simulink model "ROV_Simulation"  (R2024b) (continued)
== system ROV_Simulation/Recreate 6 DOF ref == (continued)
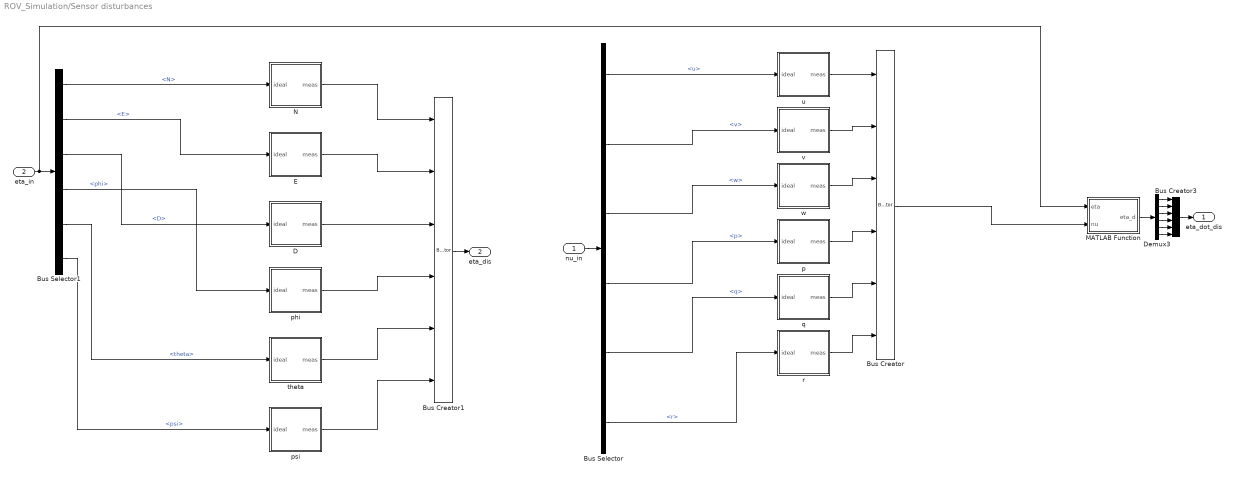
== system ROV_Simulation/Sensor disturbances ==
  nu_in : Inport
  eta_in : Inport  Port=2
  Bus Creator : BusCreator  Inputs=6  OutDataTypeStr=Bus: nu
      settings: InheritFromInputs=off
  Bus Creator1 : BusCreator  Inputs=6  OutDataTypeStr=Bus: eta
      settings: InheritFromInputs=off
  Bus Creator3 : BusCreator  Inputs=6  OutDataTypeStr=Bus: eta_d
      settings: InheritFromInputs=off
  Bus Selector : BusSelector  OutputSignals=u,v,w,p,q,r
  Bus Selector1 : BusSelector  OutputSignals=N,E,D,phi,theta,psi
  D : Subsystem
  Demux3 : Demux  Outputs=6
  E : Subsystem
  MATLAB Function : MATLAB Function  TreatAsAtomicUnit=on
  N : Subsystem
  p : Subsystem
  phi : Subsystem
  psi : Subsystem
  q : Subsystem
  r : Subsystem
  theta : Subsystem
  u : Subsystem
  v : Subsystem
  w : Subsystem
  eta_dot_dis : Outport
  eta_dis : Outport  Port=2
  wires:
  Bus Selector/6 -> r/1(ideal)  "<r>"
  Bus Selector/3 -> w/1(ideal)  "<w>"
  eta_in/1 -> MATLAB Function/1(eta), Bus Selector1/1
  Bus Selector1/2 -> E/1(ideal)  "<E>"
  Bus Selector1/3 -> D/1(ideal)  "<D>"
  Bus Selector1/4 -> phi/1(ideal)  "<phi>"
  Bus Selector1/1 -> N/1(ideal)  "<N>"
  Bus Selector/4 -> p/1(ideal)  "<p>"
  Bus Selector/2 -> v/1(ideal)  "<v>"
  Bus Selector1/6 -> psi/1(ideal)  "<psi>"
  nu_in/1 -> Bus Selector/1
  Bus Selector/5 -> q/1(ideal)  "<q>"
  Bus Selector1/5 -> theta/1(ideal)  "<theta>"
  Bus Selector/1 -> u/1(ideal)  "<u>"
  Bus Creator/1 -> MATLAB Function/2(nu)
  D/1(meas) -> Bus Creator1/3
  phi/1(meas) -> Bus Creator1/4
  theta/1(meas) -> Bus Creator1/5
  psi/1(meas) -> Bus Creator1/6
  N/1(meas) -> Bus Creator1/1
  E/1(meas) -> Bus Creator1/2
  Bus Creator1/1 -> eta_dis/1
  u/1(meas) -> Bus Creator/1
  v/1(meas) -> Bus Creator/2
  w/1(meas) -> Bus Creator/3
  p/1(meas) -> Bus Creator/4
  q/1(meas) -> Bus Creator/5
  r/1(meas) -> Bus Creator/6
  MATLAB Function/1(eta_d) -> Demux3/1
  Demux3/1 -> Bus Creator3/1
  Demux3/5 -> Bus Creator3/5
  Demux3/2 -> Bus Creator3/2
  Bus Creator3/1 -> eta_dot_dis/1
  Demux3/3 -> Bus Creator3/3
  Demux3/4 -> Bus Creator3/4
  Demux3/6 -> Bus Creator3/6

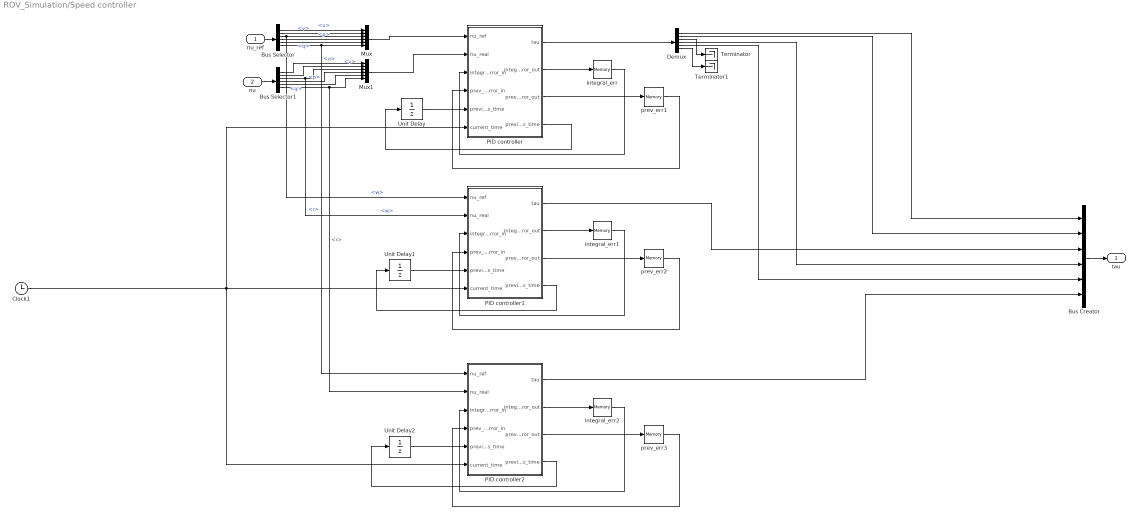
== system ROV_Simulation/Speed controller  ==
  nu_ref : Inport
  nu : Inport  Port=2
  Bus Creator : BusCreator  Inputs=6  OutDataTypeStr=Bus: tau
  Bus Selector : BusSelector  OutputSignals=u,v,w,p,q,r
  Bus Selector1 : BusSelector  OutputSignals=u,v,w,p,q,r
  Clock1 : Clock
  Demux : Demux  Outputs=6
  Mux : Mux  Inputs=6
  Mux1 : Mux  Inputs=6
  PID controller : MATLAB Function  TreatAsAtomicUnit=on
  PID controller1 : MATLAB Function  TreatAsAtomicUnit=on
  PID controller2 : MATLAB Function  TreatAsAtomicUnit=on
  Terminator : Terminator
  Terminator1 : Terminator
  Unit Delay : UnitDelay  SampleTime=-1  HasFrameUpgradeWarning=on
  Unit Delay1 : UnitDelay  SampleTime=-1  HasFrameUpgradeWarning=on
  Unit Delay2 : UnitDelay  SampleTime=-1  HasFrameUpgradeWarning=on
  integral_err : Memory
  integral_err1 : Memory
  integral_err2 : Memory
  prev_err1 : Memory
  prev_err2 : Memory
  prev_err3 : Memory
  tau : Outport
  wires:
  PID controller/1(tau) -> Demux/1
  PID controller/2(integral_error_out) -> integral_err/1
  PID controller/3(prev_error_out) -> prev_err1/1
  prev_err1/1 -> PID controller/4(prev_error_in)
  integral_err/1 -> PID controller/3(integral_error_in)
  Bus Creator/1 -> tau/1
  Demux/1 -> Bus Creator/1
  Demux/2 -> Bus Creator/2
  Demux/4 -> Bus Creator/4
  Demux/5 -> Bus Creator/5
  nu_ref/1 -> Bus Selector/1
  Mux/1 -> PID controller/1(nu_ref)
  nu/1 -> Bus Selector1/1
  Mux1/1 -> PID controller/2(nu_real)
  Unit Delay/1 -> PID controller/5("previous_time ")
  PID controller/4(previous_time) -> Unit Delay/1
  Bus Selector/1 -> Mux/1  "<u>"
  Bus Selector/2 -> Mux/2  "<v>"
  Bus Selector/4 -> Mux/4  "<p>"
  Bus Selector/5 -> Mux/5  "<q>"
  Bus Selector1/1 -> Mux1/1  "<u>"
  Bus Selector1/2 -> Mux1/2  "<v>"
  Bus Selector1/4 -> Mux1/4  "<p>"
  Bus Selector1/5 -> Mux1/5  "<q>"
  Bus Selector1/3 -> Mux1/3, PID controller1/2(nu_real)  "<w>"
  PID controller1/1(tau) -> Bus Creator/3
  PID controller1/2(integral_error_out) -> integral_err1/1
  integral_err1/1 -> PID controller1/3(integral_error_in)
  PID controller1/3(prev_error_out) -> prev_err2/1
  PID controller1/4(previous_time) -> Unit Delay1/1
  Unit Delay1/1 -> PID controller1/5("previous_time ")
  Bus Selector/3 -> Mux/3, PID controller1/1(nu_ref)  "<w>"
  prev_err2/1 -> PID controller1/4(prev_error_in)
  prev_err3/1 -> PID controller2/4(prev_error_in)
  PID controller2/1(tau) -> Bus Creator/6
  PID controller2/3(prev_error_out) -> prev_err3/1
  PID controller2/2(integral_error_out) -> integral_err2/1
  integral_err2/1 -> PID controller2/3(integral_error_in)
  PID controller2/4(previous_time) -> Unit Delay2/1
  Unit Delay2/1 -> PID controller2/5("previous_time ")
  Clock1/1 -> PID controller/6(current_time), PID controller1/6(current_time), PID controller2/6(current_time)
  Bus Selector/6 -> PID controller2/1(nu_ref), Mux/6  "<r>"
  Bus Selector1/6 -> PID controller2/2(nu_real), Mux1/6  "<r>"
  Demux/3 -> Terminator/1
  Demux/6 -> Terminator1/1

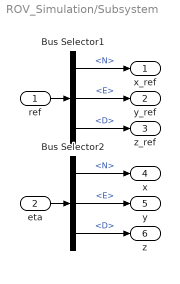
== system ROV_Simulation/Subsystem ==
  ref : Inport  OutDataTypeStr=Bus: eta
  eta : Inport  Port=2  OutDataTypeStr=Bus: eta
  Bus Selector1 : BusSelector  OutputSignals=N,E,D
  Bus Selector2 : BusSelector  OutputSignals=N,E,D
  x_ref : Outport
  y_ref : Outport  Port=2
  z_ref : Outport  Port=3
  x : Outport  Port=4
  y : Outport  Port=5
  z : Outport  Port=6
  wires:
  eta/1 -> Bus Selector2/1
  Bus Selector2/2 -> y/1  "<E>"
  ref/1 -> Bus Selector1/1
  Bus Selector2/3 -> z/1  "<D>"
  Bus Selector1/3 -> z_ref/1  "<D>"
  Bus Selector1/2 -> y_ref/1  "<E>"
  Bus Selector1/1 -> x_ref/1  "<N>"
  Bus Selector2/1 -> x/1  "<N>"

== system ROV_Simulation/Subsystem2 ==
  ref : Inport  OutDataTypeStr=Bus: eta
  eta : Inport  Port=2  OutDataTypeStr=Bus: eta
  Bus Selector1 : BusSelector  OutputSignals=phi,theta,psi
  Bus Selector2 : BusSelector  OutputSignals=phi,theta,psi
  phi_ref : Outport
  theta_ref : Outport  Port=2
  psi_ref : Outport  Port=3
  phi : Outport  Port=4
  theta : Outport  Port=5
  psi : Outport  Port=6
  wires:
  Bus Selector2/1 -> phi/1  "<phi>"
  Bus Selector1/1 -> phi_ref/1  "<phi>"
  Bus Selector1/2 -> theta_ref/1  "<theta>"
  Bus Selector1/3 -> psi_ref/1  "<psi>"
  Bus Selector2/3 -> psi/1  "<psi>"
  ref/1 -> Bus Selector1/1
  Bus Selector2/2 -> theta/1  "<theta>"
  eta/1 -> Bus Selector2/1

== system ROV_Simulation/Thruster Dynamics ==
  Tau : Inport  OutDataTypeStr=Bus: tau
  Bus Creator : BusCreator  Inputs=6  OutDataTypeStr=Bus: tau
      settings: InheritFromInputs=off
  Demux2 : Demux  Outputs=6
  Pseudo Inverse of TAM (T_inv) : Gain  Gain=T_inv  Multiplication=Matrix(K*u) (u vector)
  Saturation and dynamics : Subsystem  TreatAsAtomicUnit=on
  TAM (T) : Gain  Gain=T  Multiplication=Matrix(K*u) (u vector)
  Tau Sat : Outport  OutDataTypeStr=Bus: tau
  tag scope: tau=global
  wires:
  Bus Creator/1 -> [tau], Tau Sat/1
  Tau/1 -> Pseudo Inverse of TAM (T_inv)/1
  TAM (T)/1 -> Demux2/1
  Demux2/6 -> Bus Creator/6
  Demux2/5 -> Bus Creator/5
  Demux2/4 -> Bus Creator/4
  Demux2/3 -> Bus Creator/3
  Demux2/2 -> Bus Creator/2
  Demux2/1 -> Bus Creator/1
  Pseudo Inverse of TAM (T_inv)/1 -> Saturation and dynamics/1(In1)
  Saturation and dynamics/1(Out1) -> TAM (T)/1

== system ROV_Simulation/eta2VR ==
  eta : Inport  OutDataTypeStr=Bus: eta
  Axes to VR Axes : Subsystem
  Bus Selector12 : BusSelector  OutputSignals=N,E,D
  Bus Selector13 : BusSelector  OutputSignals=phi,theta,psi
  Euler to VR : Subsystem
  Mux : Mux  Inputs=3
  Mux1 : Mux  Inputs=3
  VR Tracer : vrlib/VR Tracer  VrmlFile=C:\Users\smaha\OneDrive\Bureau\ROVSimulator2024a\Dependencies\Animation\Good_world_BlueRov.x3d  MarkerName=Sphere  UseLines=on  UseTriads=on  MarkerScale=0.02*[1 1 1]  MarkerColorSelection=Defined as RGB values  MarkerColor=red  rgbval=[1 0 0]  Ts=1  ForceViewerOpen=off  ConnectPlane=off  PlaneCoeff=[0 1 0 0]  ConnectPoint=None  PointCoord=[0 0 0]
  VR_Rotation : Outport
  VR_Translation : Outport  Port=2
  wires:
  Bus Selector12/1 -> Mux/1  "<N>"
  Bus Selector12/2 -> Mux/2  "<E>"
  Bus Selector13/3 -> Mux1/3  "<psi>"
  Bus Selector13/2 -> Mux1/2  "<theta>"
  Axes to VR Axes/1(Xe VR) -> VR_Translation/1, VR Tracer/1
  eta/1 -> Bus Selector12/1, Bus Selector13/1
  Mux/1 -> Axes to VR Axes/1(Xe)
  Bus Selector13/1 -> Mux1/1  "<phi>"
  Bus Selector12/3 -> Mux/3  "<D>"
  Euler to VR/1(Axis Angle) -> VR_Rotation/1
  Mux1/1 -> Euler to VR/1(Euler Angles)

== system ROV_Simulation/extract 3 DOF ==
  In1 : Inport
  Bus Selector1 : BusSelector  OutputSignals=N,E,psi
  Mux : Mux  Inputs=3
  Out1 : Outport
  wires:
  Bus Selector1/1 -> Mux/1  "<N>"
  Bus Selector1/2 -> Mux/2  "<E>"
  Bus Selector1/3 -> Mux/3  "<psi>"
  In1/1 -> Bus Selector1/1
  Mux/1 -> Out1/1

== system ROV_Simulation/extract 4 DOF ==
  In1 : Inport
  Bus Selector1 : BusSelector  OutputSignals=N,E,D,psi
  Mux : Mux
  current_pos : Outport
  wires:
  Bus Selector1/1 -> Mux/1  "<N>"
  Bus Selector1/2 -> Mux/2  "<E>"
  Bus Selector1/4 -> Mux/4  "<psi>"
  In1/1 -> Bus Selector1/1
  Mux/1 -> current_pos/1
  Bus Selector1/3 -> Mux/3  "<D>"

== system ROV_Simulation/External Forces/Manual disturbance ==
  Enable : EnablePort
  Bus Creator : BusCreator  Inputs=6  OutDataTypeStr=Bus: tau
      settings: InheritFromInputs=off
  Constant : Constant  Value=EXT.Man.X
  Constant1 : Constant  Value=EXT.Man.Y
  Constant2 : Constant  Value=EXT.Man.Z
  Constant3 : Constant  Value=EXT.Man.phi
  Constant4 : Constant  Value=EXT.Man.psi
  Constant5 : Constant  Value=EXT.Man.theta
  dist : Outport  OutDataTypeStr=Bus: tau
  wires:
  Bus Creator/1 -> dist/1
  Constant3/1 -> Bus Creator/4
  Constant4/1 -> Bus Creator/6
  Constant/1 -> Bus Creator/1
  Constant5/1 -> Bus Creator/5
  Constant2/1 -> Bus Creator/3
  Constant1/1 -> Bus Creator/2

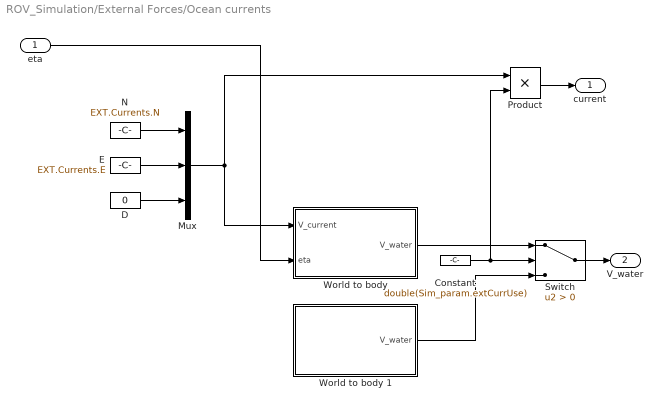
== system ROV_Simulation/External Forces/Ocean currents ==
  eta : Inport
  Constant : Constant  Value=double(Sim_param.extCurrUse)
  D : Constant  Value=0
  E : Constant  Value=EXT.Currents.E
  Mux : Mux  Inputs=3
  N : Constant  Value=EXT.Currents.N
  Product : Product
  Switch : Switch  Criteria=u2 > Threshold  SaturateOnIntegerOverflow=off  InputSameDT=off
  "World to body " : MATLAB Function  TreatAsAtomicUnit=on
  World to body 1 : MATLAB Function  TreatAsAtomicUnit=on
  current : Outport
  V_water : Outport  Port=2
  wires:
  D/1 -> Mux/3
  Mux/1 -> Product/1, "World to body "/1(V_current)
  Product/1 -> current/1
  Constant/1 -> Product/2, Switch/2
  "World to body "/1(V_water) -> Switch/1
  E/1 -> Mux/2
  World to body 1/1(V_water) -> Switch/3
  eta/1 -> "World to body "/2(eta)
  N/1 -> Mux/1
  Switch/1 -> V_water/1

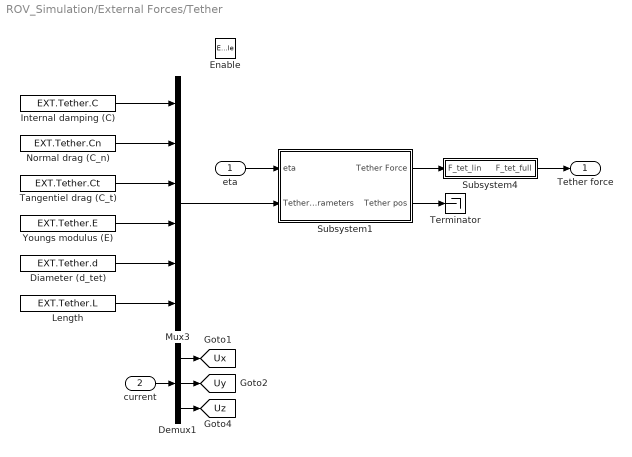
== system ROV_Simulation/External Forces/Tether ==
  eta : Inport
  current : Inport  Port=2
  Enable : EnablePort
  Demux1 : Demux  Outputs=3
  Diameter (d_tet) : Constant  Value=EXT.Tether.d
  Internal damping (C) : Constant  Value=EXT.Tether.C
  Length : Constant  Value=EXT.Tether.L
  Mux3 : Mux  Inputs=6
  Normal drag (C_n) : Constant  Value=EXT.Tether.Cn
  Subsystem1 : Subsystem
  Subsystem4 : Subsystem
  Tangentiel drag (C_t) : Constant  Value=EXT.Tether.Ct
  Terminator : Terminator
  Youngs modulus (E) : Constant  Value=EXT.Tether.E
  Tether force : Outport  OutDataTypeStr=Bus: tau
  tag scope: Ux=global, Uy=global, Uz=global
  wires:
  Demux1/1 -> [Ux]
  Youngs modulus (E)/1 -> Mux3/4
  Subsystem1/1(Tether Force) -> Subsystem4/1(F_tet_lin)
  Tangentiel drag (C_t)/1 -> Mux3/3
  Mux3/1 -> Subsystem1/2(Tether_Parameters)
  eta/1 -> Subsystem1/1(eta)
  Normal drag (C_n)/1 -> Mux3/2
  Subsystem1/2(Tether pos) -> Terminator/1
  Subsystem4/1(F_tet_full) -> Tether force/1
  current/1 -> Demux1/1
  Demux1/2 -> [Uy]
  Internal damping (C)/1 -> Mux3/1
  Demux1/3 -> [Uz]
  Length/1 -> Mux3/6
  Diameter (d_tet)/1 -> Mux3/5

== system ROV_Simulation/Sensor disturbances/D ==
  ideal : Inport
  Clock : Clock
  Constant : Constant  Value=noise(3).drift
  Delay : Delay  DelayLength=round(noise(3).delay/(1/noise(3).ts))  InitialCondition=eta_IC.D  SampleTime=1/noise(3).ts
  Product : Product
  Random Number1 : RandomNumber  Variance=noise(3).var*10^(-3)  SampleTime=1/noise(3).ts
  Sum1 : Sum  Inputs=+++
  meas : Outport
  wires:
  Random Number1/1 -> Sum1/3
  Constant/1 -> Product/2
  Sum1/1 -> Delay/1
  Clock/1 -> Product/1
  Product/1 -> Sum1/1
  ideal/1 -> Sum1/2
  Delay/1 -> meas/1

== system ROV_Simulation/Sensor disturbances/E ==
  ideal : Inport
  Clock : Clock
  Constant : Constant  Value=noise(2).drift
  Delay : Delay  DelayLength=round(noise(2).delay/(1/noise(2).ts))  InitialCondition=eta_IC.E  SampleTime=1/noise(2).ts
  Product : Product
  Random Number1 : RandomNumber  Variance=noise(2).var*10^(-3)  SampleTime=1/noise(2).ts
  Sum1 : Sum  Inputs=+++
  meas : Outport
  wires:
  Random Number1/1 -> Sum1/3
  Constant/1 -> Product/2
  Sum1/1 -> Delay/1
  Clock/1 -> Product/1
  Product/1 -> Sum1/1
  ideal/1 -> Sum1/2
  Delay/1 -> meas/1

== system ROV_Simulation/Sensor disturbances/N ==
  ideal : Inport
  Clock : Clock
  Constant : Constant  Value=noise(1).drift
  Delay : Delay  DelayLength=round(noise(1).delay/(1/noise(1).ts))  InitialCondition=eta_IC.N  SampleTime=1/noise(1).ts
  Product : Product
  Random Number1 : RandomNumber  Variance=noise(1).var*10^(-3)  SampleTime=1/noise(1).ts
  Sum1 : Sum  Inputs=+++
  meas : Outport
  wires:
  Random Number1/1 -> Sum1/3
  Constant/1 -> Product/2
  Sum1/1 -> Delay/1
  Clock/1 -> Product/1
  Product/1 -> Sum1/1
  ideal/1 -> Sum1/2
  Delay/1 -> meas/1

== system ROV_Simulation/Sensor disturbances/p ==
  ideal : Inport
  Clock : Clock
  Constant : Constant  Value=noise(10).drift
  Delay : Delay  DelayLength=round(noise(10).delay/(1/noise(10).ts))  InitialCondition=nu_IC.p  SampleTime=1/noise(10).ts
  Product : Product
  Random Number1 : RandomNumber  Variance=noise(10).var*10^(-3)  SampleTime=1/noise(10).ts
  Sum1 : Sum  Inputs=+++
  meas : Outport
  wires:
  Random Number1/1 -> Sum1/3
  Constant/1 -> Product/2
  Sum1/1 -> Delay/1
  Clock/1 -> Product/1
  Product/1 -> Sum1/1
  ideal/1 -> Sum1/2
  Delay/1 -> meas/1

== system ROV_Simulation/Sensor disturbances/phi ==
  ideal : Inport
  Clock : Clock
  Constant : Constant  Value=noise(4).drift
  Delay : Delay  DelayLength=round(noise(4).delay/(1/noise(4).ts))  InitialCondition=eta_IC.phi  SampleTime=1/noise(4).ts
  Product : Product
  Random Number1 : RandomNumber  Variance=noise(4).var*10^(-3)  SampleTime=1/noise(4).ts
  Sum1 : Sum  Inputs=+++
  meas : Outport
  wires:
  Random Number1/1 -> Sum1/3
  Constant/1 -> Product/2
  Sum1/1 -> Delay/1
  Clock/1 -> Product/1
  Product/1 -> Sum1/1
  ideal/1 -> Sum1/2
  Delay/1 -> meas/1

== system ROV_Simulation/Sensor disturbances/psi ==
  ideal : Inport
  Clock : Clock
  Constant : Constant  Value=noise(6).drift
  Delay : Delay  DelayLength=round(noise(6).delay/(1/noise(6).ts))  InitialCondition=eta_IC.psi  SampleTime=1/noise(6).ts
  Product : Product
  Random Number1 : RandomNumber  Variance=noise(6).var*10^(-3)  SampleTime=1/noise(6).ts
  Sum1 : Sum  Inputs=+++
  meas : Outport
  wires:
  Random Number1/1 -> Sum1/3
  Constant/1 -> Product/2
  Sum1/1 -> Delay/1
  Clock/1 -> Product/1
  Product/1 -> Sum1/1
  ideal/1 -> Sum1/2
  Delay/1 -> meas/1

== system ROV_Simulation/Sensor disturbances/q ==
  ideal : Inport
  Clock : Clock
  Constant : Constant  Value=noise(11).drift
  Delay : Delay  DelayLength=round(noise(11).delay/(1/noise(11).ts))  InitialCondition=nu_IC.q  SampleTime=1/noise(11).ts
  Product : Product
  Random Number1 : RandomNumber  Variance=noise(11).var*10^(-3)  SampleTime=1/noise(11).ts
  Sum1 : Sum  Inputs=+++
  meas : Outport
  wires:
  Random Number1/1 -> Sum1/3
  Constant/1 -> Product/2
  Sum1/1 -> Delay/1
  Clock/1 -> Product/1
  Product/1 -> Sum1/1
  ideal/1 -> Sum1/2
  Delay/1 -> meas/1

== system ROV_Simulation/Sensor disturbances/r ==
  ideal : Inport
  Clock : Clock
  Constant : Constant  Value=noise(12).drift
  Delay : Delay  DelayLength=round(noise(12).delay/(1/noise(12).ts))  InitialCondition=nu_IC.r  SampleTime=1/noise(12).ts
  Product : Product
  Random Number1 : RandomNumber  Variance=noise(12).var*10^(-3)  SampleTime=1/noise(12).ts
  Sum1 : Sum  Inputs=+++
  meas : Outport
  wires:
  Random Number1/1 -> Sum1/3
  Constant/1 -> Product/2
  Sum1/1 -> Delay/1
  Clock/1 -> Product/1
  Product/1 -> Sum1/1
  ideal/1 -> Sum1/2
  Delay/1 -> meas/1

== system ROV_Simulation/Sensor disturbances/theta ==
  ideal : Inport
  Clock : Clock
  Constant : Constant  Value=noise(5).drift
  Delay : Delay  DelayLength=round(noise(5).delay/(1/noise(5).ts))  InitialCondition=eta_IC.theta  SampleTime=1/noise(5).ts
  Product : Product
  Random Number1 : RandomNumber  Variance=noise(5).var*10^(-3)  SampleTime=1/noise(5).ts
  Sum1 : Sum  Inputs=+++
  meas : Outport
  wires:
  Random Number1/1 -> Sum1/3
  Constant/1 -> Product/2
  Sum1/1 -> Delay/1
  Clock/1 -> Product/1
  Product/1 -> Sum1/1
  ideal/1 -> Sum1/2
  Delay/1 -> meas/1

== system ROV_Simulation/Sensor disturbances/u ==
  ideal : Inport
  Clock : Clock
  Constant : Constant  Value=noise(7).drift
  Delay : Delay  DelayLength=round(noise(7).delay/(1/noise(7).ts))  InitialCondition=nu_IC.u  SampleTime=1/noise(7).ts
  Product : Product
  Random Number1 : RandomNumber  Variance=noise(7).var*10^(-3)  SampleTime=1/noise(7).ts
  Sum1 : Sum  Inputs=+++
  meas : Outport
  wires:
  Random Number1/1 -> Sum1/3
  Constant/1 -> Product/2
  Sum1/1 -> Delay/1
  Clock/1 -> Product/1
  Product/1 -> Sum1/1
  ideal/1 -> Sum1/2
  Delay/1 -> meas/1

== system ROV_Simulation/Sensor disturbances/v ==
  ideal : Inport
  Clock : Clock
  Constant : Constant  Value=noise(8).drift
  Delay : Delay  DelayLength=round(noise(8).delay/(1/noise(8).ts))  InitialCondition=nu_IC.v  SampleTime=1/noise(8).ts
  Product : Product
  Random Number1 : RandomNumber  Variance=noise(8).var*10^(-3)  SampleTime=1/noise(8).ts
  Sum1 : Sum  Inputs=+++
  meas : Outport
  wires:
  Random Number1/1 -> Sum1/3
  Constant/1 -> Product/2
  Sum1/1 -> Delay/1
  Clock/1 -> Product/1
  Product/1 -> Sum1/1
  ideal/1 -> Sum1/2
  Delay/1 -> meas/1

== system ROV_Simulation/Sensor disturbances/w ==
  ideal : Inport
  Clock : Clock
  Constant : Constant  Value=noise(9).drift
  Delay : Delay  DelayLength=round(noise(9).delay/(1/noise(9).ts))  InitialCondition=nu_IC.w  SampleTime=1/noise(9).ts
  Product : Product
  Random Number1 : RandomNumber  Variance=noise(9).var*10^(-3)  SampleTime=1/noise(9).ts
  Sum1 : Sum  Inputs=+++
  meas : Outport
  wires:
  Random Number1/1 -> Sum1/3
  Constant/1 -> Product/2
  Sum1/1 -> Delay/1
  Clock/1 -> Product/1
  Product/1 -> Sum1/1
  ideal/1 -> Sum1/2
  Delay/1 -> meas/1

== system ROV_Simulation/Thruster Dynamics/Saturation and dynamics ==
  In1 : Inport  Partition=on  PartitionDimension=1  PartitionWidth=1  PartitionOffset=0
  For Each : ForEach  DisableCoverage=on
  ThrusterSaturation : Saturate  UpperLimit=thrustUpperLimit  LowerLimit=thrustLowerLimit  ZeroCross=off
  Transfer Fcn14 : TransferFcn  Numerator=[6136 108700]  Denominator=[1 89 9258 108700]
  Out1 : Outport  ConcatenationDimension=1
  wires:
  Transfer Fcn14/1 -> Out1/1
  In1/1 -> ThrusterSaturation/1
  ThrusterSaturation/1 -> Transfer Fcn14/1  "tau_x"

== system ROV_Simulation/eta2VR/Axes to VR Axes ==
  Xe : Inport
  Concatenate : Concatenate  NumInputs=3
  Demux : Demux  Outputs=3
      settings: DisplayOption=none
  rad-->deg1 : Gain  Gain=-1  SaturateOnIntegerOverflow=on  OutDataTypeStr=Inherit: Same as input  ParamDataTypeStr=Inherit: Same as input
  Xe VR : Outport  VectorParamsAs1DForOutWhenUnconnected=off
  wires:
  Xe/1 -> Demux/1
  Demux/2 -> Concatenate/3
  Demux/3 -> rad-->deg1/1
  Concatenate/1 -> Xe VR/1
  rad-->deg1/1 -> Concatenate/2
  Demux/1 -> Concatenate/1

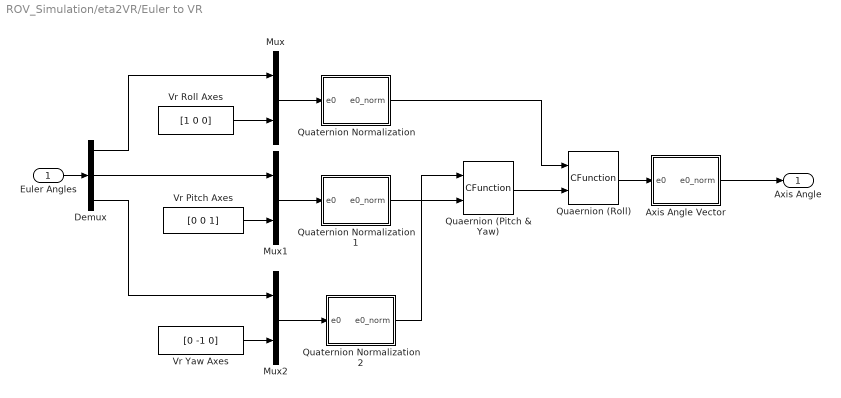
== system ROV_Simulation/eta2VR/Euler to VR ==
  Euler Angles : Inport
  Axis Angle Vector : Subsystem
  Demux : Demux  Outputs=3
      settings: DisplayOption=none
  Mux : Mux  Inputs=2
  Mux1 : Mux  Inputs=2
  Mux2 : Mux  Inputs=2
  Quaernion (Pitch & Yaw) : CFunction  OutputCode=|  GenerateCodeAsIs=off  CodegenOutputCode=|  CustomCodeSettingLocation=ModelConfigurationParameters
      OutputCode=|
      | qr[0] = q[0]*r[0] - q[1]*r[1] - q[2]*r[2] - q[3]*r[3];
      | qr[1] = q[0]*r[1] + q[1]*r[0] + q[2]*r[3] - q[3]*r[2];
      | qr[2] = q[0]*r[2] + q[2]*r[0] + q[3]*r[1] - q[1]*r[3];
      | qr[3] = q[0]*r[3] + q[3]*r[0] + q[1]*r[2] - q[2]*r[1];
      CodegenOutputCode=|
      | qr[0] = q[0]*r[0] - q[1]*r[1] - q[2]*r[2] - q[3]*r[3];
      | qr[1] = q[0]*r[1] + q[1]*r[0] + q[2]*r[3] - q[3]*r[2];
      | qr[2] = q[0]*r[2] + q[2]*r[0] + q[3]*r[1] - q[1]*r[3];
      | qr[3] = q[0]*r[3] + q[3]*r[0] + q[1]*r[2] - q[2]*r[1];
  Quaernion (Roll) : CFunction  OutputCode=|  GenerateCodeAsIs=off  CodegenOutputCode=|  CustomCodeSettingLocation=ModelConfigurationParameters
      OutputCode=|
      | qr[0] = q[0]*r[0] - q[1]*r[1] - q[2]*r[2] - q[3]*r[3];
      | qr[1] = q[0]*r[1] + q[1]*r[0] + q[2]*r[3] - q[3]*r[2];
      | qr[2] = q[0]*r[2] + q[2]*r[0] + q[3]*r[1] - q[1]*r[3];
      | qr[3] = q[0]*r[3] + q[3]*r[0] + q[1]*r[2] - q[2]*r[1];
      CodegenOutputCode=|
      | qr[0] = q[0]*r[0] - q[1]*r[1] - q[2]*r[2] - q[3]*r[3];
      | qr[1] = q[0]*r[1] + q[1]*r[0] + q[2]*r[3] - q[3]*r[2];
      | qr[2] = q[0]*r[2] + q[2]*r[0] + q[3]*r[1] - q[1]*r[3];
      | qr[3] = q[0]*r[3] + q[3]*r[0] + q[1]*r[2] - q[2]*r[1];
  Quaternion Normalization : Subsystem
  Quaternion Normalization1 : Subsystem
  Quaternion Normalization2 : Subsystem
  Vr Pitch Axes : Constant  Value=[0 0 1]
  Vr Roll Axes : Constant  Value=[1 0 0]
  Vr Yaw Axes : Constant  Value=[0 -1 0]
  Axis Angle : Outport  VectorParamsAs1DForOutWhenUnconnected=off
  wires:
  Axis Angle Vector/1(e0_norm) -> Axis Angle/1
  Quaternion Normalization2/1(e0_norm) -> Quaernion (Pitch & Yaw)/1
  Quaternion Normalization1/1(e0_norm) -> Quaernion (Pitch & Yaw)/2
  Mux2/1 -> Quaternion Normalization2/1(e0)
  Mux1/1 -> Quaternion Normalization1/1(e0)
  Mux/1 -> Quaternion Normalization/1(e0)
  Demux/3 -> Mux2/1
  Vr Yaw Axes/1 -> Mux2/2
  Demux/2 -> Mux1/1
  Vr Pitch Axes/1 -> Mux1/2
  Demux/1 -> Mux/1
  Vr Roll Axes/1 -> Mux/2
  Euler Angles/1 -> Demux/1
  Quaernion (Roll)/1 -> Axis Angle Vector/1(e0)
  Quaernion (Pitch & Yaw)/1 -> Quaernion (Roll)/2
  Quaternion Normalization/1(e0_norm) -> Quaernion (Roll)/1

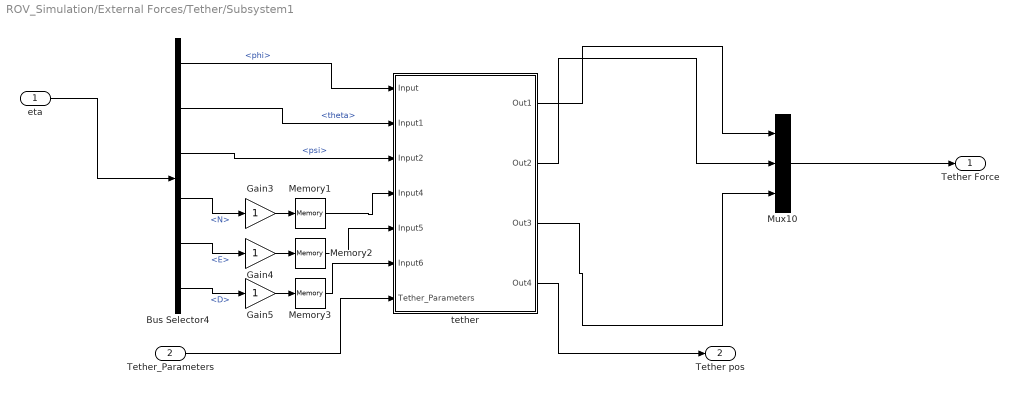
== system ROV_Simulation/External Forces/Tether/Subsystem1 ==
  eta : Inport
  Tether_Parameters : Inport  Port=2
  Bus Selector4 : BusSelector  OutputSignals=phi,theta,psi,N,E,D
  Gain3 : Gain
  Gain4 : Gain
  Gain5 : Gain
  Memory1 : Memory  InitialCondition=P10(1)
  Memory2 : Memory  InitialCondition=P10(2)
  Memory3 : Memory  InitialCondition=P10(3)
  Mux10 : Mux  Inputs=3
  tether : Subsystem
  Tether Force : Outport
  Tether pos : Outport  Port=2
  wires:
  Memory3/1 -> tether/6(Input6)
  Memory2/1 -> tether/5(Input5)
  Memory1/1 -> tether/4(Input4)
  Bus Selector4/1 -> tether/1(Input)  "<phi>"
  Bus Selector4/2 -> tether/2(Input1)  "<theta>"
  Bus Selector4/3 -> tether/3(Input2)  "<psi>"
  Tether_Parameters/1 -> tether/7(Tether_Parameters)
  Gain3/1 -> Memory1/1
  Gain4/1 -> Memory2/1
  Gain5/1 -> Memory3/1
  tether/1(Out1) -> Mux10/1
  tether/2(Out2) -> Mux10/2
  tether/3(Out3) -> Mux10/3
  Mux10/1 -> Tether Force/1
  tether/4(Out4) -> Tether pos/1
  Bus Selector4/4 -> Gain3/1  "<N>"
  Bus Selector4/5 -> Gain4/1  "<E>"
  Bus Selector4/6 -> Gain5/1  "<D>"
  eta/1 -> Bus Selector4/1

== system ROV_Simulation/External Forces/Tether/Subsystem4 ==
  F_tet_lin : Inport
  Bus Creator : BusCreator  Inputs=6  OutDataTypeStr=Bus: tau
      settings: InheritFromInputs=off
  Constant : Constant  Value=0
  Demux : Demux  Outputs=3
  F_tet_full : Outport  OutDataTypeStr=Bus: tau
  wires:
  Constant/1 -> Bus Creator/6, Bus Creator/4, Bus Creator/5
  Bus Creator/1 -> F_tet_full/1
  Demux/1 -> Bus Creator/1
  Demux/2 -> Bus Creator/2
  Demux/3 -> Bus Creator/3
  F_tet_lin/1 -> Demux/1
